simulink model "TestHarness_inf"  (R2016a) (continued)
== system TestHarness_inf/outputPinsBlock1 == (continued)
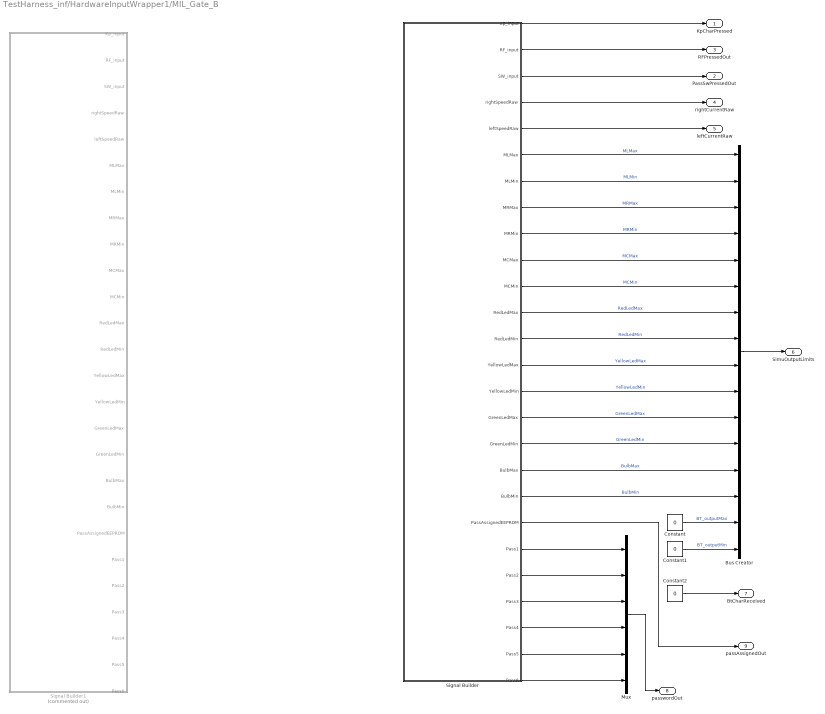
== system TestHarness_inf/HardwareInputWrapper1/MIL_Gate_B ==
  Bus Creator : BusCreator  Inputs=16
  Constant : Constant  Value=0
  Constant1 : Constant  Value=0
  Constant2 : Constant  Value=0
  Mux : Mux  Inputs=6
  Signal Builder : Subsystem  Tag=STV Subsys  LoadFcn=sigbuilder_block('load');  StartFcn=sigbuilder_block('start');  StopFcn=sigbuilder_block('stop');  ClipboardFcn=sigbuilder_block('clipboard');  mask(Sigbuilder block){}
      mask help: Simulink:masks:SigbuilderBlock_MD
      mask init:
      | if ~strcmp(get_param(bdroot(gcbh),'SimulationStatus'),'stopped') tuvar = sigbuilder_block('maskInit'); end
      settings: CopyFcn=sigbuilder_block('copy');  DeleteFcn=sigbuilder_block('delete');  ModelCloseFcn=sigbuilder_block('modelClose');  PreSaveFcn=sigbuilder_block('preSave');  PauseFcn=sigbuilder_block('pause');  ContinueFcn=sigbuilder_block('continue');  NameChangeFcn=sigbuilder_block('namechange');  OpenFcn=sigbuilder_block('open',[-1161 42 1012.8 468 ]);  CloseFcn=sigbuilder_block('close');
      mask Display: plot(0, 0, 100, 100,[2, 2, 32, 32, 2], [68, 8, 8, 68, 68],[32, 2], [38, 38], [32, 19, 2],[53, 60, 44], [32, 17, 17, 2],[16, 16, 31, 31]);txt = getActiveGroup(gcbh);text(2, 100, txt,'verticalAlignment', 'top');
  Signal Builder1 : Subsystem  Tag=STV Subsys  Commented=on  LoadFcn=sigbuilder_block('load');  StartFcn=sigbuilder_block('start');  StopFcn=sigbuilder_block('stop');  ClipboardFcn=sigbuilder_block('clipboard');  mask(Sigbuilder block){}
      mask help: Simulink:masks:SigbuilderBlock_MD
      mask init:
      | if ~strcmp(get_param(bdroot(gcbh),'SimulationStatus'),'stopped') tuvar = sigbuilder_block('maskInit'); end
      settings: CopyFcn=sigbuilder_block('copy');  DeleteFcn=sigbuilder_block('delete');  ModelCloseFcn=sigbuilder_block('modelClose');  PreSaveFcn=sigbuilder_block('preSave');  PauseFcn=sigbuilder_block('pause');  ContinueFcn=sigbuilder_block('continue');  NameChangeFcn=sigbuilder_block('namechange');  OpenFcn=sigbuilder_block('open',[-1139.4 30 1012.8 507 ]);  CloseFcn=sigbuilder_block('close');
      mask Display: plot(0, 0, 100, 100,[2, 2, 32, 32, 2], [68, 8, 8, 68, 68],[32, 2], [38, 38], [32, 19, 2],[53, 60, 44], [32, 17, 17, 2],[16, 16, 31, 31]);txt = getActiveGroup(gcbh);text(2, 100, txt,'verticalAlignment', 'top');
  KpCharPressed : Outport
  PassSwPressedOut : Outport  Port=2
  RFPressedOut : Outport  Port=3
  rightCurrentRaw : Outport  Port=4
  leftCurrentRaw : Outport  Port=5
  SimuOutputLimits : Outport  Port=6
  BtCharReceived : Outport  Port=7
  passwordOut : Outport  Port=8
  passAssignedOut : Outport  Port=9
  wires:
  Signal Builder/20(PassAssignedEEPROM) -> passAssignedOut/1
  Signal Builder/26(Pass6) -> Mux/6
  Signal Builder/25(Pass5) -> Mux/5
  Signal Builder/24(Pass4) -> Mux/4
  Signal Builder/23(Pass3) -> Mux/3
  Signal Builder/22(Pass2) -> Mux/2
  Signal Builder/21(Pass1) -> Mux/1
  Signal Builder/5(leftSpeedRaw) -> leftCurrentRaw/1
  Signal Builder/4(rightSpeedRaw) -> rightCurrentRaw/1
  Signal Builder/2(RF_input) -> RFPressedOut/1
  Signal Builder/1(Kp_input) -> KpCharPressed/1
  Mux/1 -> passwordOut/1
  Signal Builder/3(SW_input) -> PassSwPressedOut/1
  Constant2/1 -> BtCharReceived/1
  Constant1/1 -> Bus Creator/16  "BT_outputMin"
  Constant/1 -> Bus Creator/15  "BT_outputMax"
  Bus Creator/1 -> SimuOutputLimits/1
  Signal Builder/16(GreenLedMax) -> Bus Creator/11  "GreenLedMax"
  Signal Builder/15(YellowLedMin) -> Bus Creator/10  "YellowLedMin"
  Signal Builder/17(GreenLedMin) -> Bus Creator/12  "GreenLedMin"
  Signal Builder/14(YellowLedMax) -> Bus Creator/9  "YellowLedMax"
  Signal Builder/18(BulbMax) -> Bus Creator/13  "BulbMax"
  Signal Builder/13(RedLedMin) -> Bus Creator/8  "RedLedMin"
  Signal Builder/19(BulbMin) -> Bus Creator/14  "BulbMin"
  Signal Builder/12(RedLedMax) -> Bus Creator/7  "RedLedMax"
  Signal Builder/11(MCMin) -> Bus Creator/6  "MCMin"
  Signal Builder/10(MCMax) -> Bus Creator/5  "MCMax"
  Signal Builder/9(MRMin) -> Bus Creator/4  "MRMin"
  Signal Builder/8(MRMax) -> Bus Creator/3  "MRMax"
  Signal Builder/7(MLMin) -> Bus Creator/2  "MLMin"
  Signal Builder/6(MLMax) -> Bus Creator/1  "MLMax"

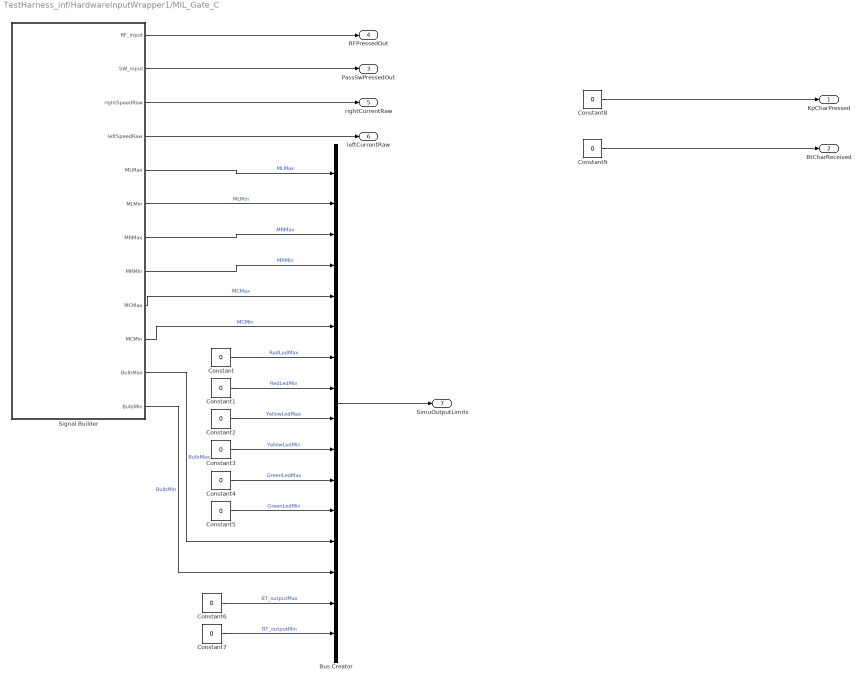
== system TestHarness_inf/HardwareInputWrapper1/MIL_Gate_C ==
  Bus Creator : BusCreator  Inputs=16
  Constant{,1..9} (10 alike) : Constant  Value=0
  Signal Builder : Subsystem  Tag=STV Subsys  LoadFcn=sigbuilder_block('load');  StartFcn=sigbuilder_block('start');  StopFcn=sigbuilder_block('stop');  ClipboardFcn=sigbuilder_block('clipboard');  mask(Sigbuilder block){}
      mask help: Simulink:masks:SigbuilderBlock_MD
      mask init:
      | if ~strcmp(get_param(bdroot(gcbh),'SimulationStatus'),'stopped') tuvar = sigbuilder_block('maskInit'); end
      settings: CopyFcn=sigbuilder_block('copy');  DeleteFcn=sigbuilder_block('delete');  ModelCloseFcn=sigbuilder_block('modelClose');  PreSaveFcn=sigbuilder_block('preSave');  PauseFcn=sigbuilder_block('pause');  ContinueFcn=sigbuilder_block('continue');  NameChangeFcn=sigbuilder_block('namechange');  OpenFcn=sigbuilder_block('open',[6 6 1012.5 507 ]);  CloseFcn=sigbuilder_block('close');
      mask Display: plot(0, 0, 100, 100,[2, 2, 32, 32, 2], [68, 8, 8, 68, 68],[32, 2], [38, 38], [32, 19, 2],[53, 60, 44], [32, 17, 17, 2],[16, 16, 31, 31]);txt = getActiveGroup(gcbh);text(2, 100, txt,'verticalAlignment', 'top');
  KpCharPressed : Outport
  BtCharReceived : Outport  Port=2
  PassSwPressedOut : Outport  Port=3
  RFPressedOut : Outport  Port=4
  rightCurrentRaw : Outport  Port=5
  leftCurrentRaw : Outport  Port=6
  SimuOutputLimits : Outport  Port=7
  wires:
  Signal Builder/4(leftSpeedRaw) -> leftCurrentRaw/1
  Signal Builder/3(rightSpeedRaw) -> rightCurrentRaw/1
  Constant7/1 -> Bus Creator/16  "BT_outputMin"
  Signal Builder/5(MLMax) -> Bus Creator/1  "MLMax"
  Signal Builder/6(MLMin) -> Bus Creator/2  "MLMin"
  Signal Builder/7(MRMax) -> Bus Creator/3  "MRMax"
  Signal Builder/8(MRMin) -> Bus Creator/4  "MRMin"
  Signal Builder/9(MCMax) -> Bus Creator/5  "MCMax"
  Signal Builder/10(MCMin) -> Bus Creator/6  "MCMin"
  Constant/1 -> Bus Creator/7  "RedLedMax"
  Constant6/1 -> Bus Creator/15  "BT_outputMax"
  Signal Builder/12(BulbMin) -> Bus Creator/14  "BulbMin"
  Constant1/1 -> Bus Creator/8  "RedLedMin"
  Signal Builder/11(BulbMax) -> Bus Creator/13  "BulbMax"
  Constant2/1 -> Bus Creator/9  "YellowLedMax"
  Constant5/1 -> Bus Creator/12  "GreenLedMin"
  Constant3/1 -> Bus Creator/10  "YellowLedMin"
  Constant4/1 -> Bus Creator/11  "GreenLedMax"
  Bus Creator/1 -> SimuOutputLimits/1
  Signal Builder/2(SW_input) -> PassSwPressedOut/1
  Signal Builder/1(RF_input) -> RFPressedOut/1
  Constant9/1 -> BtCharReceived/1
  Constant8/1 -> KpCharPressed/1

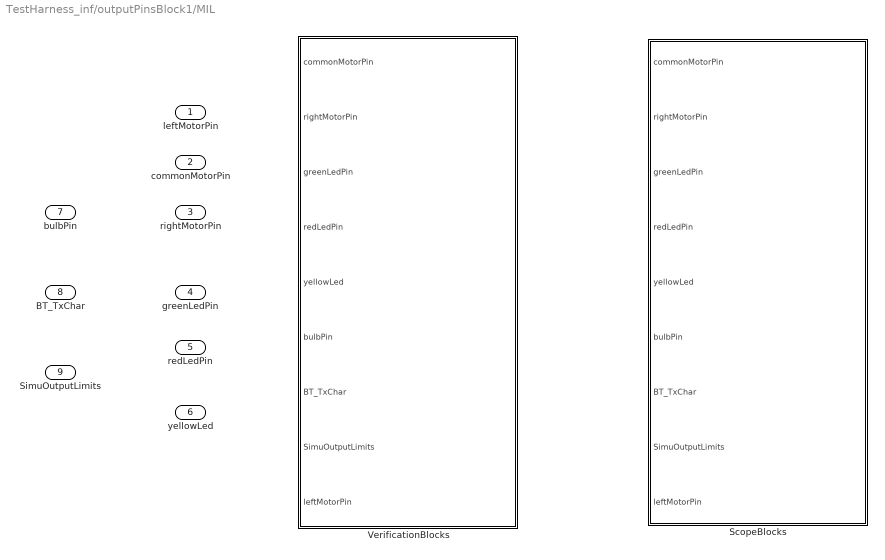
== system TestHarness_inf/outputPinsBlock1/MIL ==
  "leftMotorPin " : Inport
  "commonMotorPin " : Inport  Port=2
  "rightMotorPin " : Inport  Port=3
  greenLedPin : Inport  Port=4
  redLedPin : Inport  Port=5
  yellowLed : Inport  Port=6
  bulbPin : Inport  Port=7
  BT_TxChar : Inport  Port=8
  SimuOutputLimits : Inport  Port=9
  ScopeBlocks : Subsystem  VariantControl=VisualOutputs
  "VerificationBlocks " : Subsystem  VariantControl=VVOutputs

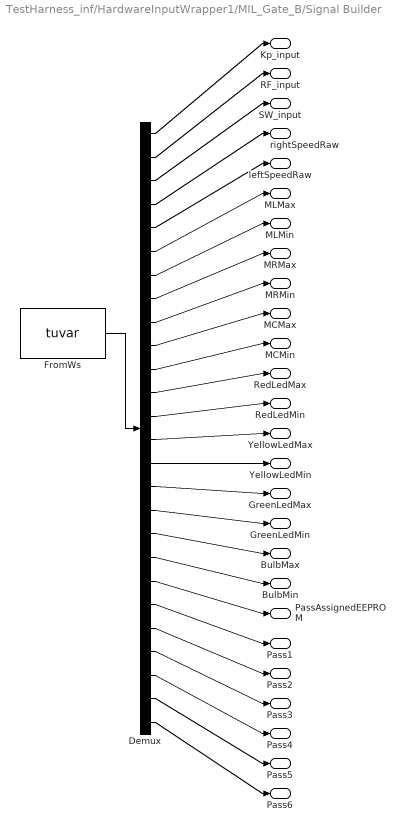
== system TestHarness_inf/HardwareInputWrapper1/MIL_Gate_B/Signal Builder ==
  Demux : Demux  Tag=STV Demux  Outputs=26
  FromWs : FromWorkspace  Tag=STV FromWs  VariableName=tuvar  SampleTime=0  OutputAfterFinalValue=Holding final value
  Kp_input : Outport  Tag=STV Outport
  RF_input : Outport  Tag=STV Outport  Port=2
  SW_input : Outport  Tag=STV Outport  Port=3
  rightSpeedRaw : Outport  Tag=STV Outport  Port=4
  leftSpeedRaw : Outport  Tag=STV Outport  Port=5
  MLMax : Outport  Tag=STV Outport  Port=6
  MLMin : Outport  Tag=STV Outport  Port=7
  MRMax : Outport  Tag=STV Outport  Port=8
  MRMin : Outport  Tag=STV Outport  Port=9
  MCMax : Outport  Tag=STV Outport  Port=10
  MCMin : Outport  Tag=STV Outport  Port=11
  RedLedMax : Outport  Tag=STV Outport  Port=12
  RedLedMin : Outport  Tag=STV Outport  Port=13
  YellowLedMax : Outport  Tag=STV Outport  Port=14
  YellowLedMin : Outport  Tag=STV Outport  Port=15
  GreenLedMax : Outport  Tag=STV Outport  Port=16
  GreenLedMin : Outport  Tag=STV Outport  Port=17
  BulbMax : Outport  Tag=STV Outport  Port=18
  BulbMin : Outport  Tag=STV Outport  Port=19
  PassAssignedEEPROM : Outport  Tag=STV Outport  Port=20
  Pass1 : Outport  Tag=STV Outport  Port=21
  Pass2 : Outport  Tag=STV Outport  Port=22
  Pass3 : Outport  Tag=STV Outport  Port=23
  Pass4 : Outport  Tag=STV Outport  Port=24
  Pass5 : Outport  Tag=STV Outport  Port=25
  Pass6 : Outport  Tag=STV Outport  Port=26
  wires:
  Demux/26 -> Pass6/1
  Demux/25 -> Pass5/1
  Demux/24 -> Pass4/1
  Demux/23 -> Pass3/1
  Demux/22 -> Pass2/1
  Demux/21 -> Pass1/1
  Demux/20 -> PassAssignedEEPROM/1
  Demux/19 -> BulbMin/1
  Demux/18 -> BulbMax/1
  Demux/17 -> GreenLedMin/1
  Demux/16 -> GreenLedMax/1
  Demux/15 -> YellowLedMin/1
  Demux/14 -> YellowLedMax/1
  Demux/13 -> RedLedMin/1
  Demux/12 -> RedLedMax/1
  Demux/11 -> MCMin/1
  Demux/10 -> MCMax/1
  Demux/9 -> MRMin/1
  Demux/8 -> MRMax/1
  Demux/7 -> MLMin/1
  Demux/6 -> MLMax/1
  Demux/5 -> leftSpeedRaw/1
  Demux/4 -> rightSpeedRaw/1
  Demux/3 -> SW_input/1
  Demux/2 -> RF_input/1
  Demux/1 -> Kp_input/1
  FromWs/1 -> Demux/1

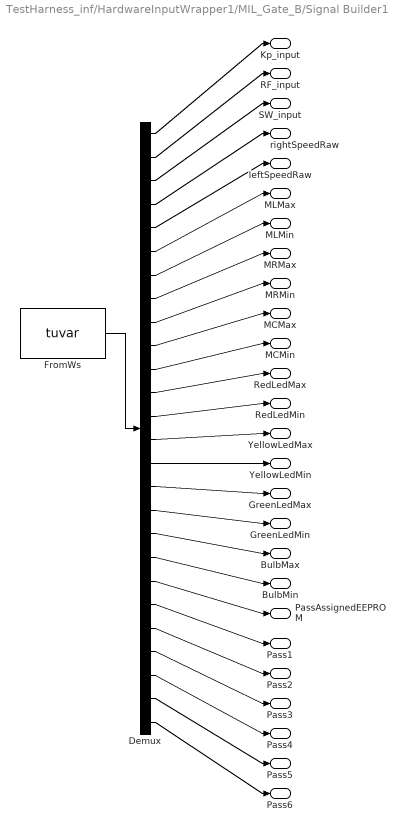
== system TestHarness_inf/HardwareInputWrapper1/MIL_Gate_B/Signal Builder1 ==
  Demux : Demux  Tag=STV Demux  Outputs=26
  FromWs : FromWorkspace  Tag=STV FromWs  VariableName=tuvar  SampleTime=0  OutputAfterFinalValue=Holding final value
  Kp_input : Outport  Tag=STV Outport
  RF_input : Outport  Tag=STV Outport  Port=2
  SW_input : Outport  Tag=STV Outport  Port=3
  rightSpeedRaw : Outport  Tag=STV Outport  Port=4
  leftSpeedRaw : Outport  Tag=STV Outport  Port=5
  MLMax : Outport  Tag=STV Outport  Port=6
  MLMin : Outport  Tag=STV Outport  Port=7
  MRMax : Outport  Tag=STV Outport  Port=8
  MRMin : Outport  Tag=STV Outport  Port=9
  MCMax : Outport  Tag=STV Outport  Port=10
  MCMin : Outport  Tag=STV Outport  Port=11
  RedLedMax : Outport  Tag=STV Outport  Port=12
  RedLedMin : Outport  Tag=STV Outport  Port=13
  YellowLedMax : Outport  Tag=STV Outport  Port=14
  YellowLedMin : Outport  Tag=STV Outport  Port=15
  GreenLedMax : Outport  Tag=STV Outport  Port=16
  GreenLedMin : Outport  Tag=STV Outport  Port=17
  BulbMax : Outport  Tag=STV Outport  Port=18
  BulbMin : Outport  Tag=STV Outport  Port=19
  PassAssignedEEPROM : Outport  Tag=STV Outport  Port=20
  Pass1 : Outport  Tag=STV Outport  Port=21
  Pass2 : Outport  Tag=STV Outport  Port=22
  Pass3 : Outport  Tag=STV Outport  Port=23
  Pass4 : Outport  Tag=STV Outport  Port=24
  Pass5 : Outport  Tag=STV Outport  Port=25
  Pass6 : Outport  Tag=STV Outport  Port=26
  wires:
  FromWs/1 -> Demux/1
  Demux/1 -> Kp_input/1
  Demux/2 -> RF_input/1
  Demux/3 -> SW_input/1
  Demux/4 -> rightSpeedRaw/1
  Demux/5 -> leftSpeedRaw/1
  Demux/6 -> MLMax/1
  Demux/7 -> MLMin/1
  Demux/8 -> MRMax/1
  Demux/9 -> MRMin/1
  Demux/10 -> MCMax/1
  Demux/11 -> MCMin/1
  Demux/12 -> RedLedMax/1
  Demux/13 -> RedLedMin/1
  Demux/14 -> YellowLedMax/1
  Demux/15 -> YellowLedMin/1
  Demux/16 -> GreenLedMax/1
  Demux/17 -> GreenLedMin/1
  Demux/18 -> BulbMax/1
  Demux/19 -> BulbMin/1
  Demux/20 -> PassAssignedEEPROM/1
  Demux/21 -> Pass1/1
  Demux/22 -> Pass2/1
  Demux/23 -> Pass3/1
  Demux/24 -> Pass4/1
  Demux/25 -> Pass5/1
  Demux/26 -> Pass6/1

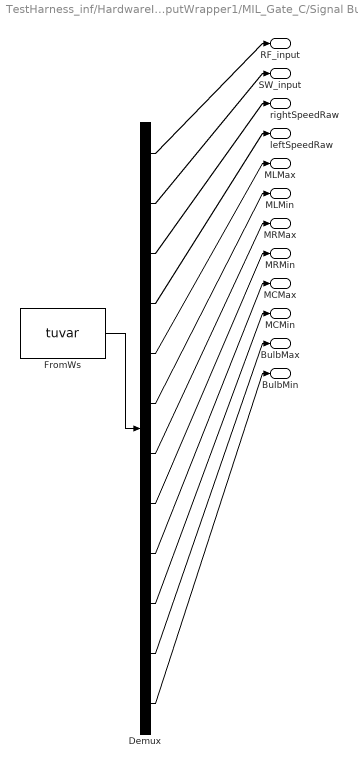
== system TestHarness_inf/HardwareInputWrapper1/MIL_Gate_C/Signal Builder ==
  Demux : Demux  Tag=STV Demux  Outputs=12
  FromWs : FromWorkspace  Tag=STV FromWs  VariableName=tuvar  SampleTime=0
      SigBuilderData=|
      | common.afterFinalStr = 'Extrapolate'
      | common.sampleTime = 0
      | dataSet(1).timeRange = [0 120]
      | dataSet(1).name = 'RFinputOnly_C'
      | dataSet(2).timeRange = [0 120]
      | dataSet(2).name = 'RF_SW_input_C'
      | dataSet(3).timeRange = [0 120]
      | dataSet(3).name = 'SW_PauseBeforeSideDelay_C'
      | dataSet(4).timeRange = [0 120]
      | dataSet(4).name = 'SW_input2Cycles_C'
      | sbobj.Groups(1).Name = 'RFinputOnly_C'
      | sbobj.Groups(1).Signals(1).Name = 'RF_input'
      | sbobj.Groups(1).Signals(1).XData = [0 3.85 3.85 4.01 4.01 31.15 31.15 31.347 31.347 120]
      | sbobj.Groups(1).Signals(1).YData = [0 0 1 1 0 0 1 1 0 0]
      | sbobj.Groups(1).Signals(2).Name = 'SW_input'
      | sbobj.Groups(1).Signals(2).XData = [0 120]
      | sbobj.Groups(1).Signals(2).YData = [0 0]
      | sbobj.Groups(1).Signals(3).Name = 'rightSpeedRaw'
      | sbobj.Groups(1).Signals(3).XData = [0 0.001 0.002 0.003 0.004 0.005 0.006 0.007 0.008 0.009 0.01 0.011 ... (60001 elements, 1x60001)]
      | sbobj.Groups(1).Signals(3).YData = [1010 1010 1010 1010 1010 1010 1010 1010 1010 1010 1010 1010 ... (60001 elements, 1x60001)]
      | sbobj.Groups(1).Signals(4).Name = 'leftSpeedRaw'
      | sbobj.Groups(1).Signals(4).XData = [0 48 48 72 72 120]
      | sbobj.Groups(1).Signals(4).YData = [0 0 1 1 0 0]
      | sbobj.Groups(1).Signals(5).Name = 'MLMax'
      | sbobj.Groups(1).Signals(5).XData = [0 3.5 3.5 19.5 19.5 31 31 35 35 120]
      | sbobj.Groups(1).Signals(5).YData = [0.1 0.1 1.1 1.1 0.1 0.1 1.1 1.1 0.1 0.1]
      | sbobj.Groups(1).Signals(6).Name = 'MLMin'
      | sbobj.Groups(1).Signals(6).XData = [0 4.05 4.05 18.5 18.5 31.5 31.5 34.25 34.25 120]
      | sbobj.Groups(1).Signals(6).YData = [-0.1 -0.1 0.9 0.9 -0.1 -0.1 0.9 0.9 -0.1 -0.1]
      | sbobj.Groups(1).Signals(7).Name = 'MRMax'
      | sbobj.Groups(1).Signals(7).XData = [0 6.75 6.75 19.5 19.5 30.35 120]
      | sbobj.Groups(1).Signals(7).YData = [0.1 0.1 1.1 1.1 0.1 0.1 0.1]
      | sbobj.Groups(1).Signals(8).Name = 'MRMin'
      | sbobj.Groups(1).Signals(8).XData = [0 7.55 7.55 18.5 18.5 120]
      | sbobj.Groups(1).Signals(8).YData = [-0.1 -0.1 0.9 0.9 -0.1 -0.1]
      | sbobj.Groups(1).Signals(9).Name = 'MCMax'
      | sbobj.Groups(1).Signals(9).XData = [0 31.9 31.9 46.2 46.2 120]
      | sbobj.Groups(1).Signals(9).YData = [1.1 1.1 0.1 0.1 1.1 1.1]
      | sbobj.Groups(1).Signals(10).Name = 'MCMin'
      | sbobj.Groups(1).Signals(10).XData = [0 31 31 47.7 47.7 120]
      | sbobj.Groups(1).Signals(10).YData = [0.9 0.9 -0.1 -0.1 0.9 0.9]
      | sbobj.Groups(1).Signals(11).Name = 'BulbMax'
      | sbobj.Groups(1).Signals(11).XData = [0 3.76 3.76 46.8 46.8 120]
      | sbobj.Groups(1).Signals(11).YData = [0.1 0.1 1.1 1.1 0.1 0.1]
      | sbobj.Groups(1).Signals(12).Name = 'BulbMin'
      | sbobj.Groups(1).Signals(12).XData = [0 4.27 4.27 46.2 46.2 120]
      | sbobj.Groups(1).Signals(12).YData = [-0.1 -0.1 0.9 0.9 -0.1 -0.1]
      | sbobj.Groups(1).TimeRange = [0 120]
      | sbobj.Groups(2).Name = 'RF_SW_input_C'
      | sbobj.Groups(2).Signals(1).Name = 'RF_input'
      | sbobj.Groups(2).Signals(1).XData = [0 3.85 3.85 4.01 4.01 120]
      | sbobj.Groups(2).Signals(1).YData = [0 0 1 1 0 0]
      | sbobj.Groups(2).Signals(2).Name = 'SW_input'
      | sbobj.Groups(2).Signals(2).XData = [0 31.17 31.17 31.65 31.65 120]
      | sbobj.Groups(2).Signals(2).YData = [0 0 1 1 0 0]
      | sbobj.Groups(2).Signals(3).Name = 'rightSpeedRaw'
      | sbobj.Groups(2).Signals(3).XData = [0 0.001 0.002 0.003 0.004 0.005 0.006 0.007 0.008 0.009 0.01 0.011 ... (60001 elements, 1x60001)]
      | sbobj.Groups(2).Signals(3).YData = [1010 1010 1010 1010 1010 1010 1010 1010 1010 1010 1010 1010 ... (60001 elements, 1x60001)]
      | sbobj.Groups(2).Signals(4).Name = 'leftSpeedRaw'
      | sbobj.Groups(2).Signals(4).XData = [0 48 48 72 72 120]
      | sbobj.Groups(2).Signals(4).YData = [0 0 1 1 0 0]
      | sbobj.Groups(2).Signals(5).Name = 'MLMax'
      | sbobj.Groups(2).Signals(5).XData = [0 3.5 3.5 19.5 19.5 31 31 35 35 120]
      | sbobj.Groups(2).Signals(5).YData = [0.1 0.1 1.1 1.1 0.1 0.1 1.1 1.1 0.1 0.1]
      | sbobj.Groups(2).Signals(6).Name = 'MLMin'
      | sbobj.Groups(2).Signals(6).XData = [0 4.05 4.05 18.5 18.5 31.5 31.5 34.25 34.25 120]
      | sbobj.Groups(2).Signals(6).YData = [-0.1 -0.1 0.9 0.9 -0.1 -0.1 0.9 0.9 -0.1 -0.1]
      | sbobj.Groups(2).Signals(7).Name = 'MRMax'
      | sbobj.Groups(2).Signals(7).XData = [0 6.75 6.75 19.5 19.5 30.35 120]
      | sbobj.Groups(2).Signals(7).YData = [0.1 0.1 1.1 1.1 0.1 0.1 0.1]
      | sbobj.Groups(2).Signals(8).Name = 'MRMin'
      | sbobj.Groups(2).Signals(8).XData = [0 7.55 7.55 18.5 18.5 120]
      | sbobj.Groups(2).Signals(8).YData = [-0.1 -0.1 0.9 0.9 -0.1 -0.1]
      | sbobj.Groups(2).Signals(9).Name = 'MCMax'
      | sbobj.Groups(2).Signals(9).XData = [0 31.9 31.9 46.2 46.2 120]
      | sbobj.Groups(2).Signals(9).YData = [1.1 1.1 0.1 0.1 1.1 1.1]
      | sbobj.Groups(2).Signals(10).Name = 'MCMin'
      | sbobj.Groups(2).Signals(10).XData = [0 31 31 47.7 47.7 120]
      | sbobj.Groups(2).Signals(10).YData = [0.9 0.9 -0.1 -0.1 0.9 0.9]
      | sbobj.Groups(2).Signals(11).Name = 'BulbMax'
      | sbobj.Groups(2).Signals(11).XData = [0 3.76 3.76 46.8 46.8 120]
      | sbobj.Groups(2).Signals(11).YData = [0.1 0.1 1.1 1.1 0.1 0.1]
      | sbobj.Groups(2).Signals(12).Name = 'BulbMin'
      | sbobj.Groups(2).Signals(12).XData = [0 4.27 4.27 46.2 46.2 120]
      | sbobj.Groups(2).Signals(12).YData = [-0.1 -0.1 0.9 0.9 -0.1 -0.1]
      | sbobj.Groups(2).TimeRange = [0 120]
      | sbobj.Groups(3).Name = 'SW_PauseBeforeSideDelay_C'
      | sbobj.Groups(3).Signals(1).Name = 'RF_input'
      | sbobj.Groups(3).Signals(1).XData = [0 120]
      | sbobj.Groups(3).Signals(1).YData = [0 0]
      | sbobj.Groups(3).Signals(2).Name = 'SW_input'
      | sbobj.Groups(3).Signals(2).XData = [0 3.88 3.88 4.55 4.55 5.5 5.5 6 6 11.35 11.35 12.1 12.1 31.17 31.17 31.65 31.65 120]
      | sbobj.Groups(3).Signals(2).YData = [0 0 1 1 0 0 1 1 0 0 1 1 0 0 1 1 0 0]
      | sbobj.Groups(3).Signals(3).Name = 'rightSpeedRaw'
      | sbobj.Groups(3).Signals(3).XData = [0 0.001 0.002 0.003 0.004 0.005 0.006 0.007 0.008 0.009 0.01 0.011 ... (60001 elements, 1x60001)]
      | sbobj.Groups(3).Signals(3).YData = [1010 1010 1010 1010 1010 1010 1010 1010 1010 1010 1010 1010 ... (60001 elements, 1x60001)]
      | sbobj.Groups(3).Signals(4).Name = 'leftSpeedRaw'
      | sbobj.Groups(3).Signals(4).XData = [0 0.001 0.002 0.003 0.004 0.005 0.006 0.007 0.008 0.009 0.01 0.011 ... (60001 elements, 1x60001)]
      | sbobj.Groups(3).Signals(4).YData = [1010 1010 1010 1010 1010 1010 1010 1010 1010 1010 1010 1010 ... (60001 elements, 1x60001)]
      | sbobj.Groups(3).Signals(5).Name = 'MLMax'
      | sbobj.Groups(3).Signals(5).XData = [0 3.5 3.5 12.5 12.5 31 31 35 35 120]
      | sbobj.Groups(3).Signals(5).YData = [0.1 0.1 1.1 1.1 0.1 0.1 1.1 1.1 0.1 0.1]
      | sbobj.Groups(3).Signals(6).Name = 'MLMin'
      | sbobj.Groups(3).Signals(6).XData = [0 4.05 4.05 10.6 10.6 31.5 31.5 34.25 34.25 120]
      | sbobj.Groups(3).Signals(6).YData = [-0.1 -0.1 0.9 0.9 -0.1 -0.1 0.9 0.9 -0.1 -0.1]
      | sbobj.Groups(3).Signals(7).Name = 'MRMax'
      | sbobj.Groups(3).Signals(7).XData = [0 6.75 6.75 12.9 12.9 30.35 120]
      | sbobj.Groups(3).Signals(7).YData = [0.1 0.1 1.1 1.1 0.1 0.1 0.1]
      | sbobj.Groups(3).Signals(8).Name = 'MRMin'
      | sbobj.Groups(3).Signals(8).XData = [0 7.55 7.55 10.4 10.4 120]
      | sbobj.Groups(3).Signals(8).YData = [-0.1 -0.1 0.9 0.9 -0.1 -0.1]
      | sbobj.Groups(3).Signals(9).Name = 'MCMax'
      | sbobj.Groups(3).Signals(9).XData = [0 31.9 31.9 46.2 46.2 120]
      | sbobj.Groups(3).Signals(9).YData = [1.1 1.1 0.1 0.1 1.1 1.1]
      | sbobj.Groups(3).Signals(10).Name = 'MCMin'
      | sbobj.Groups(3).Signals(10).XData = [0 31 31 47.7 47.7 120]
      | sbobj.Groups(3).Signals(10).YData = [0.9 0.9 -0.1 -0.1 0.9 0.9]
      | sbobj.Groups(3).Signals(11).Name = 'BulbMax'
      | sbobj.Groups(3).Signals(11).XData = [0 3.76 3.76 46.8 46.8 120]
      | sbobj.Groups(3).Signals(11).YData = [0.1 0.1 1.1 1.1 0.1 0.1]
      | sbobj.Groups(3).Signals(12).Name = 'BulbMin'
      | sbobj.Groups(3).Signals(12).XData = [0 4.27 4.27 46.2 46.2 120]
      | sbobj.Groups(3).Signals(12).YData = [-0.1 -0.1 0.9 0.9 -0.1 -0.1]
      | sbobj.Groups(3).TimeRange = [0 120]
      | sbobj.Groups(4).Name = 'SW_input2Cycles_C'
      | sbobj.Groups(4).Signals(1).Name = 'RF_input'
      | sbobj.Groups(4).Signals(1).XData = [0 23 23 23.2 23.2 120]
      | sbobj.Groups(4).Signals(1).YData = [0 0 1 1 0 0]
      | sbobj.Groups(4).Signals(2).Name = 'SW_input'
      | sbobj.Groups(4).Signals(2).XData = [0 3.85 3.85 4.1 4.1 43 43 43.88 43.88 79 79 80.14 80.14 120]
      | sbobj.Groups(4).Signals(2).YData = [0 0 1 1 0 0 1 1 0 0 1 1 0 0]
      | sbobj.Groups(4).Signals(3).Name = 'rightSpeedRaw'
      | sbobj.Groups(4).Signals(3).XData = [0 0.001 0.002 0.003 0.004 0.005 0.006 0.007 0.008 0.009 0.01 0.011 ... (120001 elements, 1x120001)]
      | sbobj.Groups(4).Signals(3).YData = [1010 1010 1010 1010 1010 1010 1010 1010 1010 1010 1010 1010 ... (120001 elements, 1x120001)]
      | sbobj.Groups(4).Signals(4).Name = 'leftSpeedRaw'
      | sbobj.Groups(4).Signals(4).XData = [0 0.001 0.002 0.003 0.004 0.005 0.006 0.007 0.008 0.009 0.01 0.011 ... (119980 elements, 1x119980)]
      | sbobj.Groups(4).Signals(4).YData = [1010 1010 1010 1010 1010 1010 1010 1010 1010 1010 1010 1010 ... (119980 elements, 1x119980)]
      | sbobj.Groups(4).Signals(5).Name = 'MLMax'
      | sbobj.Groups(4).Signals(5).XData = [0 3.5 3.5 19.5 19.5 22.5 22.5 26.5 26.5 42.5 42.5 58.5 58.5 78.5 78.5 82.5 82.5 120]
      | sbobj.Groups(4).Signals(5).YData = [0.1 0.1 1.1 1.1 0.1 0.1 1.1 1.1 0.1 0.1 1.1 1.1 0.1 0.1 1.1 1.1 0.1 0.1]
      | sbobj.Groups(4).Signals(6).Name = 'MLMin'
      | sbobj.Groups(4).Signals(6).XData = [0 4.05 4.05 18.5 18.5 23.5 23.5 25.5 25.5 43.5 43.5 57.5 57.5 79.5 79.5 81.5 81.5 120]
      | sbobj.Groups(4).Signals(6).YData = [-0.1 -0.1 0.9 0.9 -0.1 -0.1 0.9 0.9 -0.1 -0.1 0.9 0.9 -0.1 -0.1 0.9 0.9 -0.1 -0.1]
      | sbobj.Groups(4).Signals(7).Name = 'MRMax'
      | sbobj.Groups(4).Signals(7).XData = [0 6.75 6.75 19.5 19.5 45.5 45.5 58.5 58.5 120]
      | sbobj.Groups(4).Signals(7).YData = [0.1 0.1 1.1 1.1 0.1 0.1 1.1 1.1 0.1 0.1]
      | sbobj.Groups(4).Signals(8).Name = 'MRMin'
      | sbobj.Groups(4).Signals(8).XData = [0 7.55 7.55 18.5 18.5 46.5 46.5 57.5 57.5 120]
      | sbobj.Groups(4).Signals(8).YData = [-0.1 -0.1 0.9 0.9 -0.1 -0.1 0.9 0.9 -0.1 -0.1]
      | sbobj.Groups(4).Signals(9).Name = 'MCMax'
      | sbobj.Groups(4).Signals(9).XData = [0 23.5 23.5 37.5 37.5 79.5 79.5 93.5 93.5 120]
      | sbobj.Groups(4).Signals(9).YData = [1.1 1.1 0.1 0.1 1.1 1.1 0.1 0.1 1.1 1.1]
      | sbobj.Groups(4).Signals(10).Name = 'MCMin'
      | sbobj.Groups(4).Signals(10).XData = [0 22.5 22.5 38.5 38.5 78.5 78.5 94.5 94.5 120]
      | sbobj.Groups(4).Signals(10).YData = [0.9 0.9 -0.1 -0.1 0.9 0.9 -0.1 -0.1 0.9 0.9]
      | sbobj.Groups(4).Signals(11).Name = 'BulbMax'
      | sbobj.Groups(4).Signals(11).XData = [0 3.76 3.76 38.9 38.9 42.5 42.5 94.5 94.5 120]
      | sbobj.Groups(4).Signals(11).YData = [0.1 0.1 1.1 1.1 0.1 0.1 1.1 1.1 0.1 0.1]
      | sbobj.Groups(4).Signals(12).Name = 'BulbMin'
      | sbobj.Groups(4).Signals(12).XData = [0 4.27 4.27 37.9 37.9 43.5 43.5 93.5 93.5 120]
      | sbobj.Groups(4).Signals(12).YData = [-0.1 -0.1 0.9 0.9 -0.1 -0.1 0.9 0.9 -0.1 -0.1]
      | sbobj.Groups(4).TimeRange = [0 120]
  RF_input : Outport  Tag=STV Outport
  SW_input : Outport  Tag=STV Outport  Port=2
  rightSpeedRaw : Outport  Tag=STV Outport  Port=3
  leftSpeedRaw : Outport  Tag=STV Outport  Port=4
  MLMax : Outport  Tag=STV Outport  Port=5
  MLMin : Outport  Tag=STV Outport  Port=6
  MRMax : Outport  Tag=STV Outport  Port=7
  MRMin : Outport  Tag=STV Outport  Port=8
  MCMax : Outport  Tag=STV Outport  Port=9
  MCMin : Outport  Tag=STV Outport  Port=10
  BulbMax : Outport  Tag=STV Outport  Port=11
  BulbMin : Outport  Tag=STV Outport  Port=12
  wires:
  Demux/12 -> BulbMin/1
  Demux/11 -> BulbMax/1
  Demux/10 -> MCMin/1
  Demux/9 -> MCMax/1
  Demux/8 -> MRMin/1
  Demux/7 -> MRMax/1
  Demux/6 -> MLMin/1
  Demux/5 -> MLMax/1
  Demux/4 -> leftSpeedRaw/1
  Demux/3 -> rightSpeedRaw/1
  Demux/2 -> SW_input/1
  Demux/1 -> RF_input/1
  FromWs/1 -> Demux/1

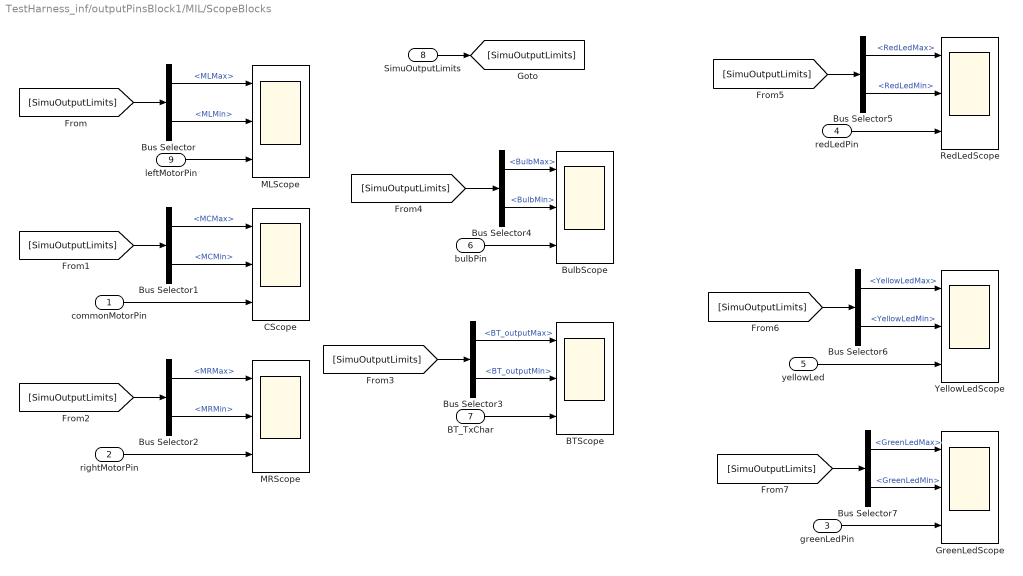
== system TestHarness_inf/outputPinsBlock1/MIL/ScopeBlocks ==
  commonMotorPin : Inport
  rightMotorPin : Inport  Port=2
  greenLedPin : Inport  Port=3
  redLedPin : Inport  Port=4
  yellowLed : Inport  Port=5
  bulbPin : Inport  Port=6
  BT_TxChar : Inport  Port=7
  SimuOutputLimits : Inport  Port=8
  leftMotorPin : Inport  Port=9
  BTScope : Scope  NumInputPorts=3
  BulbScope : Scope  NumInputPorts=3
  Bus Selector : BusSelector  OutputSignals=MLMax,MLMin
  Bus Selector1 : BusSelector  OutputSignals=MCMax,MCMin
  Bus Selector2 : BusSelector  OutputSignals=MRMax,MRMin
  Bus Selector3 : BusSelector  OutputSignals=BT_outputMax,BT_outputMin
  Bus Selector4 : BusSelector  OutputSignals=BulbMax,BulbMin
  Bus Selector5 : BusSelector  OutputSignals=RedLedMax,RedLedMin
  Bus Selector6 : BusSelector  OutputSignals=YellowLedMax,YellowLedMin
  Bus Selector7 : BusSelector  OutputSignals=GreenLedMax,GreenLedMin
  CScope : Scope  NumInputPorts=3
  GreenLedScope : Scope  NumInputPorts=3
  MLScope : Scope  NumInputPorts=3
  MRScope : Scope  NumInputPorts=3
  RedLedScope : Scope  NumInputPorts=3
  YellowLedScope : Scope  NumInputPorts=3
  wires:
  Bus Selector7/2 -> GreenLedScope/2  "<GreenLedMin>"
  Bus Selector7/1 -> GreenLedScope/1  "<GreenLedMax>"
  [SimuOutputLimits] -> Bus Selector7/1
  Bus Selector6/2 -> YellowLedScope/2  "<YellowLedMin>"
  Bus Selector6/1 -> YellowLedScope/1  "<YellowLedMax>"
  [SimuOutputLimits] -> Bus Selector6/1
  Bus Selector5/2 -> RedLedScope/2  "<RedLedMin>"
  Bus Selector5/1 -> RedLedScope/1  "<RedLedMax>"
  [SimuOutputLimits] -> Bus Selector5/1
  Bus Selector4/2 -> BulbScope/2  "<BulbMin>"
  Bus Selector4/1 -> BulbScope/1  "<BulbMax>"
  [SimuOutputLimits] -> Bus Selector4/1
  Bus Selector3/2 -> BTScope/2  "<BT_outputMin>"
  Bus Selector3/1 -> BTScope/1  "<BT_outputMax>"
  [SimuOutputLimits] -> Bus Selector3/1
  Bus Selector2/2 -> MRScope/2  "<MRMin>"
  Bus Selector2/1 -> MRScope/1  "<MRMax>"
  [SimuOutputLimits] -> Bus Selector2/1
  Bus Selector1/2 -> CScope/2  "<MCMin>"
  Bus Selector1/1 -> CScope/1  "<MCMax>"
  [SimuOutputLimits] -> Bus Selector1/1
  Bus Selector/2 -> MLScope/2  "<MLMin>"
  Bus Selector/1 -> MLScope/1  "<MLMax>"
  leftMotorPin/1 -> MLScope/3
  [SimuOutputLimits] -> Bus Selector/1
  SimuOutputLimits/1 -> [SimuOutputLimits]
  BT_TxChar/1 -> BTScope/3
  redLedPin/1 -> RedLedScope/3
  yellowLed/1 -> YellowLedScope/3
  greenLedPin/1 -> GreenLedScope/3
  bulbPin/1 -> BulbScope/3
  commonMotorPin/1 -> CScope/3
  rightMotorPin/1 -> MRScope/3

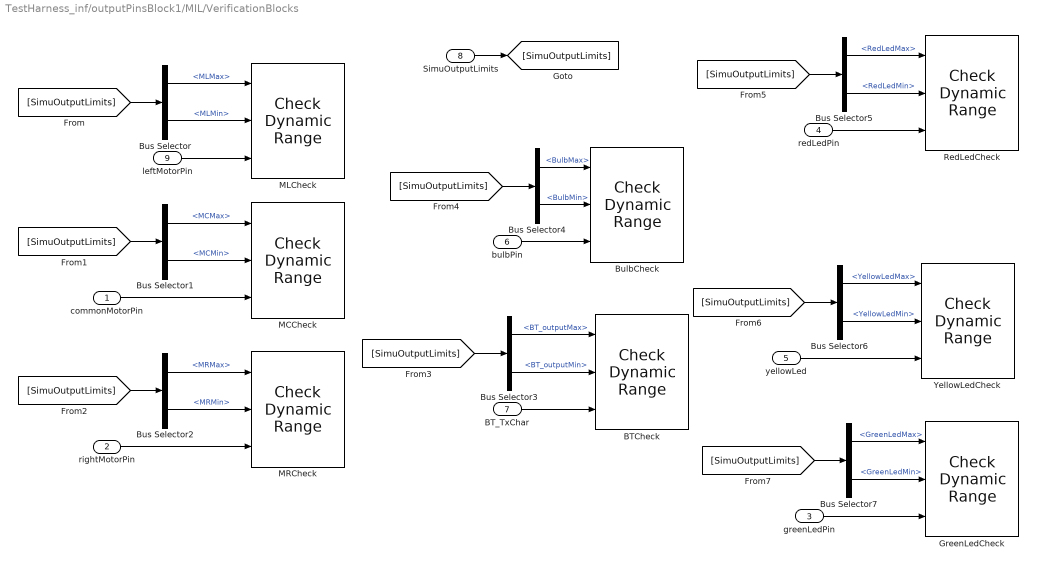
== system TestHarness_inf/outputPinsBlock1/MIL/VerificationBlocks  ==
  commonMotorPin : Inport
  rightMotorPin : Inport  Port=2
  greenLedPin : Inport  Port=3
  redLedPin : Inport  Port=4
  yellowLed : Inport  Port=5
  bulbPin : Inport  Port=6
  BT_TxChar : Inport  Port=7
  SimuOutputLimits : Inport  Port=8
  leftMotorPin : Inport  Port=9
  BTCheck : simulink/Model Verification/Check  Dynamic Range  Description=min < sig < max  enabled=on  stopWhenAssertionFail=on  export=off  icon=graphic
  BulbCheck : simulink/Model Verification/Check  Dynamic Range  Description=min < sig < max  enabled=on  stopWhenAssertionFail=on  export=off  icon=graphic
  Bus Selector : BusSelector  OutputSignals=MLMax,MLMin
  Bus Selector1 : BusSelector  OutputSignals=MCMax,MCMin
  Bus Selector2 : BusSelector  OutputSignals=MRMax,MRMin
  Bus Selector3 : BusSelector  OutputSignals=BT_outputMax,BT_outputMin
  Bus Selector4 : BusSelector  OutputSignals=BulbMax,BulbMin
  Bus Selector5 : BusSelector  OutputSignals=RedLedMax,RedLedMin
  Bus Selector6 : BusSelector  OutputSignals=YellowLedMax,YellowLedMin
  Bus Selector7 : BusSelector  OutputSignals=GreenLedMax,GreenLedMin
  GreenLedCheck : simulink/Model Verification/Check  Dynamic Range  Description=min < sig < max  enabled=on  stopWhenAssertionFail=on  export=off  icon=graphic
  MCCheck : simulink/Model Verification/Check  Dynamic Range  Description=min < sig < max  enabled=on  stopWhenAssertionFail=on  export=off  icon=graphic
  MLCheck : simulink/Model Verification/Check  Dynamic Range  Description=min < sig < max  enabled=on  stopWhenAssertionFail=on  export=off  icon=graphic
  MRCheck : simulink/Model Verification/Check  Dynamic Range  Description=min < sig < max  enabled=on  stopWhenAssertionFail=on  export=off  icon=graphic
  RedLedCheck : simulink/Model Verification/Check  Dynamic Range  Description=min < sig < max  enabled=on  stopWhenAssertionFail=on  export=off  icon=graphic
  YellowLedCheck : simulink/Model Verification/Check  Dynamic Range  Description=min < sig < max  enabled=on  stopWhenAssertionFail=on  export=off  icon=graphic
  wires:
  Bus Selector7/2 -> GreenLedCheck/2  "<GreenLedMin>"
  Bus Selector7/1 -> GreenLedCheck/1  "<GreenLedMax>"
  [SimuOutputLimits] -> Bus Selector7/1
  Bus Selector6/2 -> YellowLedCheck/2  "<YellowLedMin>"
  Bus Selector6/1 -> YellowLedCheck/1  "<YellowLedMax>"
  [SimuOutputLimits] -> Bus Selector6/1
  Bus Selector5/2 -> RedLedCheck/2  "<RedLedMin>"
  Bus Selector5/1 -> RedLedCheck/1  "<RedLedMax>"
  [SimuOutputLimits] -> Bus Selector5/1
  Bus Selector4/2 -> BulbCheck/2  "<BulbMin>"
  Bus Selector4/1 -> BulbCheck/1  "<BulbMax>"
  [SimuOutputLimits] -> Bus Selector4/1
  Bus Selector3/2 -> BTCheck/2  "<BT_outputMin>"
  Bus Selector3/1 -> BTCheck/1  "<BT_outputMax>"
  [SimuOutputLimits] -> Bus Selector3/1
  Bus Selector2/2 -> MRCheck/2  "<MRMin>"
  Bus Selector2/1 -> MRCheck/1  "<MRMax>"
  [SimuOutputLimits] -> Bus Selector2/1
  Bus Selector1/2 -> MCCheck/2  "<MCMin>"
  Bus Selector1/1 -> MCCheck/1  "<MCMax>"
  [SimuOutputLimits] -> Bus Selector1/1
  Bus Selector/2 -> MLCheck/2  "<MLMin>"
  Bus Selector/1 -> MLCheck/1  "<MLMax>"
  leftMotorPin/1 -> MLCheck/3
  [SimuOutputLimits] -> Bus Selector/1
  SimuOutputLimits/1 -> [SimuOutputLimits]
  BT_TxChar/1 -> BTCheck/3
  redLedPin/1 -> RedLedCheck/3
  yellowLed/1 -> YellowLedCheck/3
  greenLedPin/1 -> GreenLedCheck/3
  bulbPin/1 -> BulbCheck/3
  commonMotorPin/1 -> MCCheck/3
  rightMotorPin/1 -> MRCheck/3

== stateflow chart TestHarness_inf/Subsystem/SquareWaveCreatorRight ==
  state BulbOn
    entry:
    movingCounter = 0;
    during:
    movingCounter++;
    state Off
      entry:
      counter = 0;
      rightSpeedRaw_UINT16 = 0;
      du:
      counter++;
    state On
      entry:
      counter = 0;
      rightSpeedRaw_UINT16 = 1023;
      du:
      counter++;
    (default) -> Off
    On -> Off : [counter >= 100]/counter = 0;
    Off -> On : [counter >= 100]/counter = 0;
  state BulbOff
    entry:
    rightSpeedRaw_UINT16 = 0;
  (default) -> BulbOff
  BulbOff -> BulbOn : [hasChanged(ControlSignal_BOOL_in)...
      | && ControlSignal_BOOL_in]
  BulbOn -> BulbOff [order 1] : [movingCounter >= 15000]
  BulbOn -> BulbOff [order 2] : [!ControlSignal_BOOL_in]
  data: ControlSignal_BOOL_in input; rightSpeedRaw_UINT16 output uint16; counter local uint16; movingCounter local double

== stateflow chart TestHarness_inf/Subsystem/Chart ==
  (default) -> (junction 7)
  (junction 7) -> (junction 9) [order 1] : [!rightMotorPin && CommonMotorPin && !leftMotorPin]
  (junction 7) -> (junction 11) [order 2]
  (junction 9) -> (junction 10) : {leftStopped = true;
      | leftClosing = false;
      | leftOpening = false;
      | rightStopped = true;
      | rightClosing = false;
      | rightOpening = false;}
  (junction 11) -> (junction 12) [order 1] : [!rightMotorPin && CommonMotorPin && leftMotorPin]
  (junction 12) -> (junction 13) : {leftStopped = false;
      | leftClosing = false;
      | leftOpening = true;
      | rightStopped = true;
      | rightClosing = false;
      | rightOpening = false;}
  (junction 11) -> (junction 8) [order 2]
  (junction 8) -> (junction 26) [order 1] : [rightMotorPin && CommonMotorPin && !leftMotorPin]
  (junction 26) -> (junction 30) : {leftStopped = true;
      | leftClosing = false;
      | leftOpening = false;
      | rightStopped = false;
      | rightClosing = false;
      | rightOpening = true;}
  (junction 8) -> (junction 27) [order 2]
  (junction 27) -> (junction 29) [order 1] : [rightMotorPin && CommonMotorPin && leftMotorPin]
  (junction 27) -> (junction 42) [order 2]
  (junction 29) -> (junction 31) : {leftStopped = false;
      | leftClosing = false;
      | leftOpening = true;
      | rightStopped = false;
      | rightClosing = false;
      | rightOpening = true;}
  (junction 42) -> (junction 43) [order 1] : [rightMotorPin && !CommonMotorPin && leftMotorPin]
  (junction 42) -> (junction 46) [order 2]
  (junction 43) -> (junction 44) : {leftStopped = true;
      | leftClosing = false;
      | leftOpening = false;
      | rightStopped = true;
      | rightClosing = false;
      | rightOpening = false;}
  (junction 46) -> (junction 47) [order 1] : [rightMotorPin && !CommonMotorPin && !leftMotorPin]
  (junction 46) -> (junction 48) [order 2]
  (junction 47) -> (junction 45) : {leftStopped = false;
      | leftClosing = true;
      | leftOpening = false;
      | rightStopped = true;
      | rightClosing = false;
      | rightOpening = false;}
  (junction 48) -> (junction 49) [order 1] : [!rightMotorPin && !CommonMotorPin && leftMotorPin]
  (junction 48) -> (junction 33) [order 2]
  (junction 49) -> (junction 50) : {leftStopped = true;
      | leftClosing = false;
      | leftOpening = false;
      | rightStopped = false;
      | rightClosing = true;
      | rightOpening = false;}
  (junction 33) -> (junction 34) [order 1] : [!rightMotorPin && !CommonMotorPin && !leftMotorPin]
  (junction 33) -> (junction 32) [order 2]
  (junction 34) -> (junction 35) : {leftStopped = false;
      | leftClosing = true;
      | leftOpening = false;
      | rightStopped = false;
      | rightClosing = true;
      | rightOpening = false;}
  (junction 35) -> (junction 32)
  (junction 50) -> (junction 35)
  (junction 45) -> (junction 50)
  (junction 44) -> (junction 45)
  (junction 13) -> (junction 30)
  (junction 31) -> (junction 44)
  (junction 10) -> (junction 13)
  (junction 30) -> (junction 31)
  data: leftMotorPin input; CommonMotorPin input; rightMotorPin input; leftStopped output boolean; leftClosing output boolean; leftOpening output boolean; rightStopped output boolean; rightClosing output boolean; rightOpening output boolean

== stateflow chart TestHarness_inf/Subsystem/SquareWaveCreatorLeft ==
  state BulbOn
    entry:
    movingCounter = 0;
    during:
    movingCounter++;
    state Off
      entry:
      counter = 0;
      leftSpeedRaw_UINT16 = 0;
      du:
      counter++;
    state On
      entry:
      counter = 0;
      leftSpeedRaw_UINT16 = 1023;
      du:
      counter++;
    (default) -> Off
    On -> Off : [counter >= 100]/counter = 0;
    Off -> On : [counter >= 100]/counter = 0;
  state BulbOff
    entry:
    leftSpeedRaw_UINT16 = 0;
  (default) -> BulbOff
  BulbOff -> BulbOn : [hasChanged(ControlSignal_BOOL_in)...
      | && ControlSignal_BOOL_in]
  BulbOn -> BulbOff [order 1] : [movingCounter >= 15000]
  BulbOn -> BulbOff [order 2] : [!ControlSignal_BOOL_in]
  data: ControlSignal_BOOL_in input; leftSpeedRaw_UINT16 output uint16; counter local uint16; movingCounter local double
